simulink model "PlanarModelandControl"  (R2026a) (continued)
== system PlanarModelandControl/Planta/Dinamica do Veículo/Slip Angle == (continued)
  Dead Zone3/1 -> Sum6/1
  Gain10/1 -> Sum6/2
  Dead Zone4/1 -> Sum8/1
  Gain11/1 -> Sum8/2
  Sum5/1 -> Out Bus Element1/1  "FR"
  Atan2/1 -> Out Bus Element2/1  "RL"

== system PlanarModelandControl/Planta/Movimento do Veiculo/Movimento X ==
  note: Drag
  note: Movimento X
  Bus Element In1 : Inport  PortName=Yaw  Element=v_yaw
  BusElementIn2 : Inport  Port=2  PortName=Dinamica  Element=RollingResistances.Frx_RL
  BusElementIn3 : Inport  Port=2  PortName=Dinamica  Element=RollingResistances.Frx_RR
  BusElementIn4 : Inport  Port=2  PortName=Dinamica  Element=LatAndLongForces.Fx_RL
  BusElementIn5 : Inport  Port=2  PortName=Dinamica  Element=LatAndLongForces.Fx_RR
  BusElementIn : Inport  Port=3  PortName=ProjectedFrontForces  Element=Fxref_FL
  BusElementIn6 : Inport  Port=3  PortName=ProjectedFrontForces  Element=Fxref_FR
  Vy_cg : Inport  Port=4
  Gain1 : Gain  Gain=1/M
  Gain8 : Gain  Gain=0.5*rhoar*A*Cd
  Integrator : DiscreteIntegrator  InitialCondition=Vx_init  SampleTime=-1  ICPrevOutput=DiscIntNeverNeededParam  ICPrevScaledInput=DiscIntNeverNeededParam  mask(DiscretizedDiscreteIntegrator){}
      mask init:
      | %zoh
  Product : Product
  Square : Math  Operator=square
  Sum3 : Sum  Inputs=|++
  Sum4 : Sum  Inputs=|+--
  Sum5 : Sum  Inputs=|++
  Sum7 : Sum  Inputs=|++++
  Ax_cg : Outport
  Vx_cg : Outport  Port=2
  wires:
  Gain1/1 -> Ax_cg/1, Sum5/1  "Ax_Cg"
  Sum7/1 -> Sum4/1  "Forças de Tração"
  BusElementIn2/1 -> Sum3/1
  Sum3/1 -> Sum4/2  "resistivas"
  BusElementIn/1 -> Sum7/1
  Vy_cg/1 -> Product/1
  Product/1 -> Sum5/2  "yaw"
  BusElementIn6/1 -> Sum7/2
  Sum4/1 -> Gain1/1
  BusElementIn5/1 -> Sum7/4
  BusElementIn4/1 -> Sum7/3
  BusElementIn3/1 -> Sum3/2
  Gain8/1 -> Sum4/3
  Integrator/1 -> Square/1, Vx_cg/1  "Vx_cg"
  Bus Element In1/1 -> Product/2
  Sum5/1 -> Integrator/1  "Ax"
  Square/1 -> Gain8/1

== system PlanarModelandControl/Planta/Movimento do Veiculo/Movimento Y ==
  note: Movimento Y
  In Bus Element : Inport  PortName=ProjectedFrontForces  Element=Fyref_FL
  In Bus Element1 : Inport  PortName=ProjectedFrontForces  Element=Fyref_FR
  BusElementIn : Inport  Port=2  PortName=Dinamica  Element=LatAndLongForces.Fy_RL
  BusElementIn1 : Inport  Port=2  PortName=Dinamica  Element=LatAndLongForces.Fy_RR
  Bus Element In2 : Inport  Port=3  PortName=Yaw  Element=v_yaw
  Vx_cg : Inport  Port=4
  Dead Zone : DeadZone  LowerValue=-0.1  UpperValue=0.1
  Gain : Gain  Gain=1/M
  Integrator1 : DiscreteIntegrator  SampleTime=-1  ICPrevOutput=DiscIntNeverNeededParam  ICPrevScaledInput=DiscIntNeverNeededParam  mask(DiscretizedDiscreteIntegrator){}
      mask init:
      | %zoh
  Product1 : Product
  Sum : Sum  Inputs=|++++
  Sum6 : Sum  Inputs=|+-
  Ay_cg : Outport
  Vy_cg : Outport  Port=2
  wires:
  Sum/1 -> Gain/1
  In Bus Element1/1 -> Sum/2
  In Bus Element/1 -> Sum/1
  BusElementIn1/1 -> Sum/4
  BusElementIn/1 -> Sum/3
  Dead Zone/1 -> Product1/2
  Product1/1 -> Sum6/2  "Yaw"
  Integrator1/1 -> Vy_cg/1  "Vy_cg"
  Sum6/1 -> Integrator1/1  "Ay_cg"
  Vx_cg/1 -> Product1/1
  Gain/1 -> Sum6/1, Ay_cg/1  "Ay_cg"
  Bus Element In2/1 -> Dead Zone/1

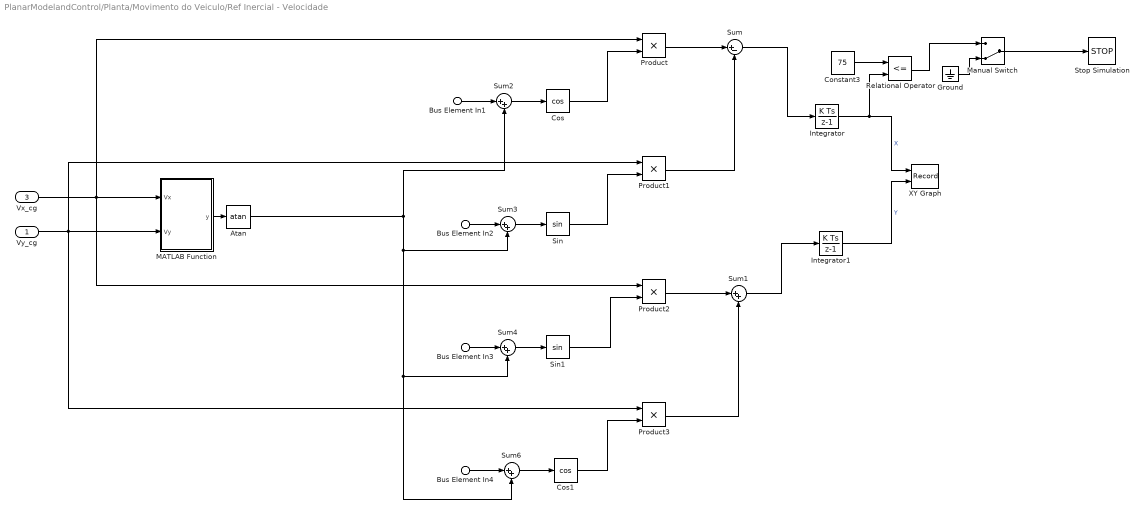
== system PlanarModelandControl/Planta/Movimento do Veiculo/Ref Inercial - Velocidade ==
  Vy_cg : Inport
  Bus Element In{1..4} (4 alike) : Inport  Port=2  PortName=Yaw  Element=s_yaw
  Vx_cg : Inport  Port=3
  Atan : Trigonometry  Operator=atan
  Constant3 : Constant  Value=75
  Cos : Trigonometry  Operator=cos
  Cos1 : Trigonometry  Operator=cos
  Ground : Ground
  Integrator : DiscreteIntegrator  SampleTime=-1  ICPrevOutput=DiscIntNeverNeededParam  ICPrevScaledInput=DiscIntNeverNeededParam  mask(DiscretizedDiscreteIntegrator){}
      mask init:
      | %zoh
  Integrator1 : DiscreteIntegrator  SampleTime=-1  ICPrevOutput=DiscIntNeverNeededParam  ICPrevScaledInput=DiscIntNeverNeededParam  mask(DiscretizedDiscreteIntegrator){}
      mask init:
      | %zoh
  MATLAB Function : MATLAB Function  TreatAsAtomicUnit=on
  Manual Switch : ManualSwitch  CurrentSetting=0
  Product{,1..3} (4 alike) : Product
  Relational Operator : RelationalOperator  Operator=<=  OutDataTypeStr=boolean  RndMeth=Simplest  InputSameDT=off
  Sin : Trigonometry
  Sin1 : Trigonometry
  Stop Simulation : Stop
  Sum : Sum  Inputs=|+-
  Sum{1..4,6} (5 alike) : Sum  Inputs=|++
  XY Graph : Record  NumPorts=2.0  FileSettingsInternal="{\"entries\":[{\"content\":{\"excelSettings\":{\"content\":{},\"type\":\"Streamout.ExcelSettings\"},\"parquetSettings\":{\"content\":{},\"type\":\"Streamout.ParquetSettings\"}},\"type\":\"Streamout.FileSettingStorage\",\"uuid\":\"\"}],\"packageUris\":[\"http:\\/\\/schema.mathworks.com\\/mf0\\/streamout_blocks\\/R2020a\"],\"version\":\"1.0\"}"  FrameSettings=[0, 0]  Layout=[1 1]  SignalsInternal="{\"entries\":[{\"content\":{\"signals\":[{\"content\":{\"blockPath\":[],\"dimensions\":[1],\"domain\":\"\",\"lineColor\":\"#7e2f8e\",\"lineWidth\":2,\"port\":1,\"sid\":[\"\"],\"signalID\":1,\"signalName\":\"X\"},\"type\":\"RecordBlkView.Signal\",\"uuid\":\"\"},{\"content\":{\"blockPath\":[],\"dimensions\":[1],\"domain\":\"\",\"lineColor\":\"#77ac30\",\"lineWidth\":2,\"port\":2,\"sid\":[\"\"],\"signalID\":2,\"signalName\":\"Y\"},\"type\":\"RecordBlkView.Signal\",\"uuid\":\"\"}]},\"type\":\"RecordBlkView.InputSignals\",\"uuid\":\"\"}],\"packageUris\":[\"http:\\/\\/schema.mathworks.com\\/mf0\\/streamout_blocks\\/R2020a\"],\"version\":\"1.0\"}"  VisualizationBindings="{\"xyplotplugin\":{\"info\":[{\"series\":[],\"subplotID\":1}]}}"  CompatibilityTag=XY  st=-1
  wires:
  Cos/1 -> Product/2
  Product/1 -> Sum/1
  Sin/1 -> Product1/2
  Product1/1 -> Sum/2
  Sum/1 -> Integrator/1
  Sin1/1 -> Product2/2
  Product2/1 -> Sum1/1
  Product3/1 -> Sum1/2
  Cos1/1 -> Product3/2
  Sum1/1 -> Integrator1/1
  Integrator1/1 -> XY Graph/2  "Y"
  Integrator/1 -> Relational Operator/2, XY Graph/1  "X"
  Bus Element In1/1 -> Sum2/1
  Bus Element In2/1 -> Sum3/1
  Bus Element In3/1 -> Sum4/1
  Bus Element In4/1 -> Sum6/1
  Vx_cg/1 -> Product/1, Product2/1, MATLAB Function/1(Vx)
  Vy_cg/1 -> Product3/1, Product1/1, MATLAB Function/2(Vy)
  MATLAB Function/1(y) -> Atan/1
  Sum2/1 -> Cos/1
  Sum3/1 -> Sin/1
  Atan/1 -> Sum2/2, Sum6/2, Sum4/2, Sum3/2
  Sum6/1 -> Cos1/1
  Sum4/1 -> Sin1/1
  Constant3/1 -> Relational Operator/1
  Relational Operator/1 -> Manual Switch/1
  Ground/1 -> Manual Switch/2
  Manual Switch/1 -> Stop Simulation/1

== system PlanarModelandControl/Planta/Movimento do Veiculo/Xres ==
  note: FR
  note: FL
  BusElementIn2 : Inport  PortName=Dinamica  Element=RollingResistances.Frx_FL
  BusElementIn3 : Inport  PortName=Dinamica  Element=RollingResistances.Frx_FR
  BusElementIn : Inport  PortName=Dinamica  Element=LatAndLongForces.Fx_FL
  BusElementIn4 : Inport  PortName=Dinamica  Element=LatAndLongForces.Fy_FL
  BusElementIn5 : Inport  PortName=Dinamica  Element=LatAndLongForces.Fy_FR
  BusElementIn1 : Inport  PortName=Dinamica  Element=LatAndLongForces.Fx_FR
  Bus Element In3 : Inport  Port=2  PortName=Driver  Element=steering_outer
  Bus Element In1 : Inport  Port=2  PortName=Driver  Element=steering_inner
  Bus Element In2 : Inport  Port=2  PortName=Driver  Element=steering_inner
  Cos : Trigonometry  Operator=cos
  Cos1 : Trigonometry
  Cos2 : Trigonometry  Operator=cos
  Cos3 : Trigonometry
  Product{,1..3} (4 alike) : Product
  Sum{,1..3} (4 alike) : Sum  Inputs=|+-
  Fxref_FL : Outport
  Fxref_FR : Outport  Port=2
  wires:
  BusElementIn2/1 -> Sum/2
  Sum/1 -> Product/1
  Cos/1 -> Product/2
  Bus Element In1/1 -> Cos/1
  Product/1 -> Sum1/1
  Product1/1 -> Sum1/2
  Bus Element In2/1 -> Cos1/1
  BusElementIn4/1 -> Product1/1
  BusElementIn5/1 -> Product3/1
  Cos3/1 -> Product3/2
  Bus Element In3/1 -> Cos3/1, Cos2/1
  Product3/1 -> Sum3/2
  Product2/1 -> Sum3/1
  Cos2/1 -> Product2/2
  Sum2/1 -> Product2/1
  BusElementIn3/1 -> Sum2/2
  Cos1/1 -> Product1/2
  BusElementIn/1 -> Sum/1
  BusElementIn1/1 -> Sum2/1
  Sum1/1 -> Fxref_FL/1
  Sum3/1 -> Fxref_FR/1

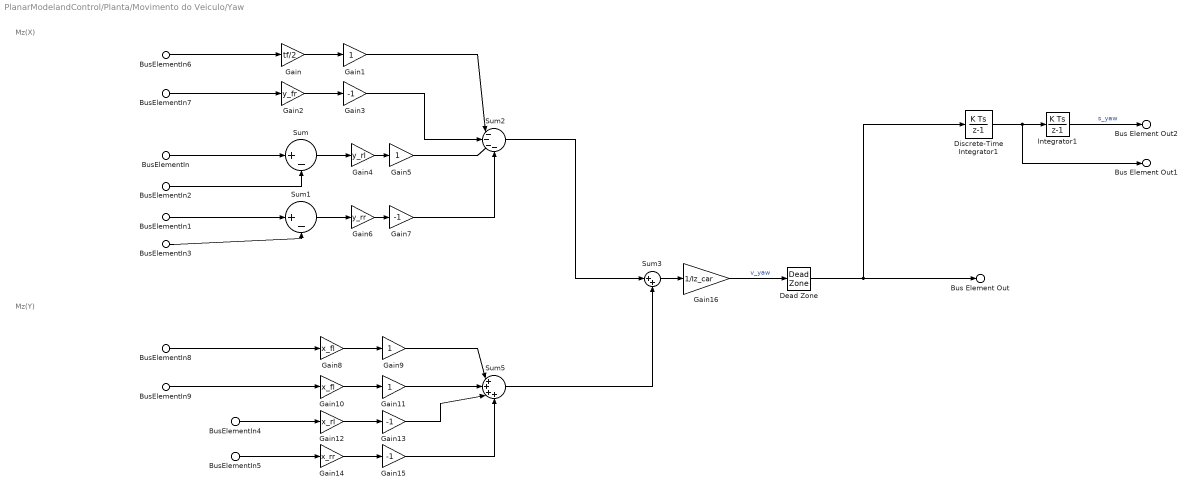
== system PlanarModelandControl/Planta/Movimento do Veiculo/Yaw ==
  note: Mz(X)
  note: Mz(Y)
  BusElementIn2 : Inport  PortName=Dinamica  Element=RollingResistances.Frx_RL
  BusElementIn3 : Inport  PortName=Dinamica  Element=RollingResistances.Frx_RR
  BusElementIn : Inport  PortName=Dinamica  Element=LatAndLongForces.Fx_RL
  BusElementIn1 : Inport  PortName=Dinamica  Element=LatAndLongForces.Fx_RR
  BusElementIn4 : Inport  PortName=Dinamica  Element=LatAndLongForces.Fy_RL
  BusElementIn5 : Inport  PortName=Dinamica  Element=LatAndLongForces.Fy_RR
  BusElementIn6 : Inport  Port=2  PortName=ProjectedFrontForces  Element=Fxref_FL
  BusElementIn7 : Inport  Port=2  PortName=ProjectedFrontForces  Element=Fxref_FR
  BusElementIn8 : Inport  Port=2  PortName=ProjectedFrontForces  Element=Fyref_FL
  BusElementIn9 : Inport  Port=2  PortName=ProjectedFrontForces  Element=Fyref_FR
  Dead Zone : DeadZone  LowerValue=-0.1  UpperValue=0.1
  Discrete-Time Integrator1 : DiscreteIntegrator  SampleTime=-1  ICPrevOutput=DiscIntNeverNeededParam  ICPrevScaledInput=DiscIntNeverNeededParam
  Gain : Gain  Gain=tf/2
  Gain{1,5,9,11} (4 alike) : Gain
  Gain10 : Gain  Gain=x_fl
  Gain12 : Gain  Gain=x_rl
  Gain{3,7,13,15} (4 alike) : Gain  Gain=-1
  Gain14 : Gain  Gain=x_rr
  Gain16 : Gain  Gain=1/Iz_car
  Gain2 : Gain  Gain=y_fr
  Gain4 : Gain  Gain=y_rl
  Gain6 : Gain  Gain=y_rr
  Gain8 : Gain  Gain=x_fl
  Integrator1 : DiscreteIntegrator  SampleTime=-1  ICPrevOutput=DiscIntNeverNeededParam  ICPrevScaledInput=DiscIntNeverNeededParam  mask(DiscretizedDiscreteIntegrator){}
      mask init:
      | %zoh
  Sum : Sum  Inputs=|+-
  Sum1 : Sum  Inputs=|+-
  Sum2 : Sum  Inputs=|----
  Sum3 : Sum  Inputs=|++
  Sum5 : Sum  Inputs=|++++
  Bus Element Out : Outport  VectorParamsAs1DForOutWhenUnconnected=off  PortName=Yaw  Element=a_yaw
  Bus Element Out1 : Outport  VectorParamsAs1DForOutWhenUnconnected=off  PortName=Yaw  Element=v_yaw
  Bus Element Out2 : Outport  VectorParamsAs1DForOutWhenUnconnected=off  PortName=Yaw  Element=s_yaw
  wires:
  BusElementIn3/1 -> Sum1/2
  BusElementIn2/1 -> Sum/2
  Gain/1 -> Gain1/1
  Gain2/1 -> Gain3/1
  Sum/1 -> Gain4/1
  Gain4/1 -> Gain5/1
  Sum1/1 -> Gain6/1
  Gain6/1 -> Gain7/1
  Gain1/1 -> Sum2/1
  Gain3/1 -> Sum2/2
  Gain5/1 -> Sum2/3
  Gain15/1 -> Sum5/4
  Gain13/1 -> Sum5/3
  Gain11/1 -> Sum5/2
  Gain9/1 -> Sum5/1
  Gain14/1 -> Gain15/1
  Gain12/1 -> Gain13/1
  Gain10/1 -> Gain11/1
  Gain8/1 -> Gain9/1
  Sum2/1 -> Sum3/1
  Sum5/1 -> Sum3/2
  Sum3/1 -> Gain16/1
  Gain16/1 -> Dead Zone/1  "v_yaw"
  Integrator1/1 -> Bus Element Out2/1  "s_yaw"
  BusElementIn/1 -> Sum/1
  BusElementIn1/1 -> Sum1/1
  BusElementIn5/1 -> Gain14/1
  BusElementIn4/1 -> Gain12/1
  Gain7/1 -> Sum2/4
  Discrete-Time Integrator1/1 -> Integrator1/1, Bus Element Out1/1
  Dead Zone/1 -> Bus Element Out/1, Discrete-Time Integrator1/1
  BusElementIn6/1 -> Gain/1
  BusElementIn7/1 -> Gain2/1
  BusElementIn8/1 -> Gain8/1
  BusElementIn9/1 -> Gain10/1

== system PlanarModelandControl/Planta/Movimento do Veiculo/Yres ==
  note: FL
  note: FL
  Bus Element In1 : Inport  PortName=Driver  Element=steering_inner
  Bus Element In2 : Inport  PortName=Driver  Element=steering_outer
  BusElementIn : Inport  Port=2  PortName=Dinamica  Element=RollingResistances.Frx_FL
  BusElementIn1 : Inport  Port=2  PortName=Dinamica  Element=RollingResistances.Frx_FR
  BusElementIn2 : Inport  Port=2  PortName=Dinamica  Element=LatAndLongForces.Fx_FL
  BusElementIn3 : Inport  Port=2  PortName=Dinamica  Element=LatAndLongForces.Fx_FR
  BusElementIn4 : Inport  Port=2  PortName=Dinamica  Element=LatAndLongForces.Fy_FL
  BusElementIn5 : Inport  Port=2  PortName=Dinamica  Element=LatAndLongForces.Fy_FR
  Cos : Trigonometry
  Cos1 : Trigonometry  Operator=cos
  Cos2 : Trigonometry
  Cos3 : Trigonometry  Operator=cos
  Product{,1..3} (4 alike) : Product
  Sum : Sum  Inputs=|+-
  Sum1 : Sum  Inputs=|++
  Sum2 : Sum  Inputs=|+-
  Sum3 : Sum  Inputs=|++
  Fyref_FL : Outport
  Fyref_FR : Outport  Port=2
  wires:
  Product1/1 -> Sum1/2
  Cos1/1 -> Product1/2
  Bus Element In1/1 -> Cos1/1, Cos/1
  Sum/1 -> Product/1
  Cos/1 -> Product/2
  Product/1 -> Sum1/1
  Product2/1 -> Sum3/1
  Cos2/1 -> Product2/2
  Sum2/1 -> Product2/1
  BusElementIn1/1 -> Sum2/2
  Bus Element In2/1 -> Cos3/1, Cos2/1
  Cos3/1 -> Product3/2
  Product3/1 -> Sum3/2
  BusElementIn4/1 -> Product1/1
  BusElementIn5/1 -> Product3/1
  BusElementIn3/1 -> Sum2/1
  Sum3/1 -> Fyref_FR/1
  Sum1/1 -> Fyref_FL/1
  BusElementIn/1 -> Sum/2
  BusElementIn2/1 -> Sum/1

== system PlanarModelandControl/Controle/Direct Yaw Control/MDYC/Feedback ==
  note: Limite inf e sup do motor
  Yaw rate : Inport
  Vx : Inport  Port=2
  Yaw_des : Inport  Port=3
  Pedal map : Inport  Port=4
  Gain : Gain  Gain=Ts
  KI : Product
  KP : Product
  Limite min : Lookup_n-D  NumberOfTableDimensions=1  BreakpointsForDimension1=pedal  BreakpointsForDimension3=[5, 7]  Table=Mz_minF  InternalRulePriority=Speed  RndMeth=Simplest
  Limite máx : Lookup_n-D  NumberOfTableDimensions=1  BreakpointsForDimension1=pedal  BreakpointsForDimension3=[5, 7]  Table=Mz_maxF  InternalRulePriority=Speed  RndMeth=Simplest
  Saturation Dynamic : sl_discontinuities/Saturation Dynamic  OutMin=[]  OutMax=[]  OutDataTypeStr=Inherit: Same as second input  OutDataType=fixdt(1, 16)  OutScaling=2^-10  DoSatur=off
  Sum : Sum  Inputs=|+-
  Sum1 : Sum  Inputs=|++
  Sum2 : Sum  Inputs=|++
  Unit Delay : UnitDelay  SampleTime=-1
  n-D Lookup Table : Lookup_n-D  NumberOfTableDimensions=1  BreakpointsForDimension1=Vx_axis  BreakpointsForDimension3=[5, 7]  Table=Kp_data  InternalRulePriority=Speed  RndMeth=Simplest
  n-D Lookup Table1 : Lookup_n-D  NumberOfTableDimensions=1  BreakpointsForDimension1=Vx_axis  BreakpointsForDimension3=[5, 7]  Table=Ki_data  InternalRulePriority=Speed  RndMeth=Simplest
  Mz_FB : Outport
  wires:
  Pedal map/1 -> Limite min/1, Limite máx/1
  Limite máx/1 -> Saturation Dynamic/1
  Limite min/1 -> Saturation Dynamic/3
  Yaw_des/1 -> Sum/1  "Yaw_Des"
  Yaw rate/1 -> Sum/2  "Yaw_rate"
  n-D Lookup Table/1 -> KP/2
  n-D Lookup Table1/1 -> KI/2
  Sum/1 -> KI/1, KP/1
  KI/1 -> Gain/1
  Gain/1 -> Sum1/2  "Ki"
  Unit Delay/1 -> Sum1/1
  Sum1/1 -> Sum2/2, Unit Delay/1
  KP/1 -> Sum2/1
  Vx/1 -> n-D Lookup Table1/1, n-D Lookup Table/1
  Sum2/1 -> Saturation Dynamic/2
  Saturation Dynamic/1 -> Mz_FB/1

== system PlanarModelandControl/Controle/Direct Yaw Control/MDYC/Feedfoward ==
  note: Limite inf e sup do motor
  CornR : Inport
  CornF : Inport  Port=2
  K delta : Inport  Port=3
  Ay : Inport  Port=4
  Pedal map : Inport  Port=5
  Add : Sum
  Divide : Product  Inputs=*/
  Gain : Gain  Gain=2*L
  Limite min : Lookup_n-D  NumberOfTableDimensions=1  BreakpointsForDimension1=pedal  BreakpointsForDimension3=[5, 7]  Table=Mz_minF  InternalRulePriority=Speed  RndMeth=Simplest
  Limite máx : Lookup_n-D  NumberOfTableDimensions=1  BreakpointsForDimension1=pedal  BreakpointsForDimension3=[5, 7]  Table=Mz_maxF  InternalRulePriority=Speed  RndMeth=Simplest
  Product : Product
  Product1 : Product
  Product2 : Product
  Saturation Dynamic : sl_discontinuities/Saturation Dynamic  OutMin=[]  OutMax=[]  OutDataTypeStr=Inherit: Same as second input  OutDataType=fixdt(1, 16)  OutScaling=2^-10  DoSatur=off
  Mz_FF : Outport
  wires:
  Limite min/1 -> Saturation Dynamic/3
  Pedal map/1 -> Limite min/1, Limite máx/1
  Limite máx/1 -> Saturation Dynamic/1
  CornR/1 -> Add/1, Product/1
  CornF/1 -> Product/2, Add/2
  Product/1 -> Gain/1
  Gain/1 -> Divide/1
  Add/1 -> Divide/2
  Divide/1 -> Product1/1
  K delta/1 -> Product1/2
  Product1/1 -> Product2/1
  Product2/1 -> Saturation Dynamic/2
  Saturation Dynamic/1 -> Mz_FF/1
  Ay/1 -> Product2/2

== system PlanarModelandControl/Controle/Direct Yaw Control/ON//OFF/If Action Subsystem ==
  Mz_ajustado : Inport
  Action Port : ActionPort  ActionPortLabel=if(u1<0.05 | u2<1 | u3<2 | u4>0)
  Gain : Gain  Gain=0
  Mz_OFF : Outport
  wires:
  Mz_ajustado/1 -> Gain/1
  Gain/1 -> Mz_OFF/1

== system PlanarModelandControl/Controle/Direct Yaw Control/ON//OFF/If Action Subsystem1 ==
  Mz_ajustado : Inport
  Action Port : ActionPort  ActionPortLabel=else
  Mz_ON : Outport
  wires:
  Mz_ajustado/1 -> Mz_ON/1

== system PlanarModelandControl/Controle/Direct Yaw Control/Yaw desejado/ref < limit ==
  Yaw_ref : Inport
  Action Port : ActionPort  ActionPortLabel=else
  Yaw_des : Outport
  wires:
  Yaw_ref/1 -> Yaw_des/1

== system PlanarModelandControl/Controle/Direct Yaw Control/Yaw desejado/ref > limit ==
  Yaw_limit : Inport
  Yaw_ref : Inport  Port=2
  Action Port : ActionPort  ActionPortLabel=if(u2 > u1)
  Product : Product
  Sign : Signum
  Yaw_des : Outport
  wires:
  Yaw_ref/1 -> Sign/1
  Yaw_limit/1 -> Product/1
  Sign/1 -> Product/2
  Product/1 -> Yaw_des/1

== system PlanarModelandControl/Controle/Traction Control/TC_FL/Fuzzy Controller ==
  wheel slip : Inport
  Discrete Derivative1 : simulink/Discrete/Discrete Derivative  gainval=1.0  ICPrevScaledInput=0.0  OutMin=[]  OutMax=[]  OutDataType=fixdt(1, 16)  OutScaling=2^-10  DoSatur=off
  Fuzzy Logic Controller : fuzblock/Fuzzy Logic  Controller  FIS='tc6.fis'  OutputSampleNumber=101  DataType=double  UseLookupTable=off  NumBreakpoints=10  FuzzifiedInputs=off  RuleFiringStrengths=off  RuleOutputs=off  AggregatedOutputs=off  SimulateUsing=Code generation  OutOfRangeInputValueMessage=warning  NoRuleFiredMessage=warning  EmptyOutputFuzzySetMessage=warning
  Mux : Mux  Inputs=2
  Saturation : Saturate  UpperLimit=1  LowerLimit=0
  Torque reducing coefficient : Outport
  wires:
  Mux/1 -> Fuzzy Logic Controller/1
  Fuzzy Logic Controller/1 -> Saturation/1
  Saturation/1 -> Torque reducing coefficient/1
  Discrete Derivative1/1 -> Mux/2
  wheel slip/1 -> Mux/1, Discrete Derivative1/1

== system PlanarModelandControl/Controle/Traction Control/TC_FR/Fuzzy Controller ==
  wheel slip : Inport
  Discrete Derivative1 : simulink/Discrete/Discrete Derivative  gainval=1.0  ICPrevScaledInput=0.0  OutMin=[]  OutMax=[]  OutDataType=fixdt(1, 16)  OutScaling=2^-10  DoSatur=off
  Fuzzy Logic Controller : fuzblock/Fuzzy Logic  Controller  FIS='tc6.fis'  OutputSampleNumber=101  DataType=double  UseLookupTable=off  NumBreakpoints=10  FuzzifiedInputs=off  RuleFiringStrengths=off  RuleOutputs=off  AggregatedOutputs=off  SimulateUsing=Code generation  OutOfRangeInputValueMessage=warning  NoRuleFiredMessage=warning  EmptyOutputFuzzySetMessage=warning
  Mux : Mux  Inputs=2
  Saturation : Saturate  UpperLimit=1  LowerLimit=0
  Torque reducing coefficient : Outport
  wires:
  Mux/1 -> Fuzzy Logic Controller/1
  Fuzzy Logic Controller/1 -> Saturation/1
  Saturation/1 -> Torque reducing coefficient/1
  Discrete Derivative1/1 -> Mux/2
  wheel slip/1 -> Mux/1, Discrete Derivative1/1

== system PlanarModelandControl/Controle/Traction Control/TC_RL/Fuzzy Controller ==
  wheel slip : Inport
  Discrete Derivative1 : simulink/Discrete/Discrete Derivative  gainval=1.0  ICPrevScaledInput=0.0  OutMin=[]  OutMax=[]  OutDataType=fixdt(1, 16)  OutScaling=2^-10  DoSatur=off
  Fuzzy Logic Controller : fuzblock/Fuzzy Logic  Controller  FIS='tc6.fis'  OutputSampleNumber=101  DataType=double  UseLookupTable=off  NumBreakpoints=10  FuzzifiedInputs=off  RuleFiringStrengths=off  RuleOutputs=off  AggregatedOutputs=off  SimulateUsing=Code generation  OutOfRangeInputValueMessage=warning  NoRuleFiredMessage=warning  EmptyOutputFuzzySetMessage=warning
  Mux : Mux  Inputs=2
  Saturation : Saturate  UpperLimit=1  LowerLimit=0
  Torque reducing coefficient : Outport
  wires:
  Mux/1 -> Fuzzy Logic Controller/1
  Fuzzy Logic Controller/1 -> Saturation/1
  Saturation/1 -> Torque reducing coefficient/1
  Discrete Derivative1/1 -> Mux/2
  wheel slip/1 -> Mux/1, Discrete Derivative1/1

== system PlanarModelandControl/Controle/Traction Control/TC_RR/Fuzzy Controller ==
  wheel slip : Inport
  Discrete Derivative1 : simulink/Discrete/Discrete Derivative  gainval=1.0  ICPrevScaledInput=0.0  OutMin=[]  OutMax=[]  OutDataType=fixdt(1, 16)  OutScaling=2^-10  DoSatur=off
  Fuzzy Logic Controller : fuzblock/Fuzzy Logic  Controller  FIS='tc6.fis'  OutputSampleNumber=101  DataType=double  UseLookupTable=off  NumBreakpoints=10  FuzzifiedInputs=off  RuleFiringStrengths=off  RuleOutputs=off  AggregatedOutputs=off  SimulateUsing=Code generation  OutOfRangeInputValueMessage=warning  NoRuleFiredMessage=warning  EmptyOutputFuzzySetMessage=warning
  Mux : Mux  Inputs=2
  Saturation : Saturate  UpperLimit=1  LowerLimit=0
  Torque reducing coefficient : Outport
  wires:
  Mux/1 -> Fuzzy Logic Controller/1
  Fuzzy Logic Controller/1 -> Saturation/1
  Saturation/1 -> Torque reducing coefficient/1
  Discrete Derivative1/1 -> Mux/2
  wheel slip/1 -> Mux/1, Discrete Derivative1/1

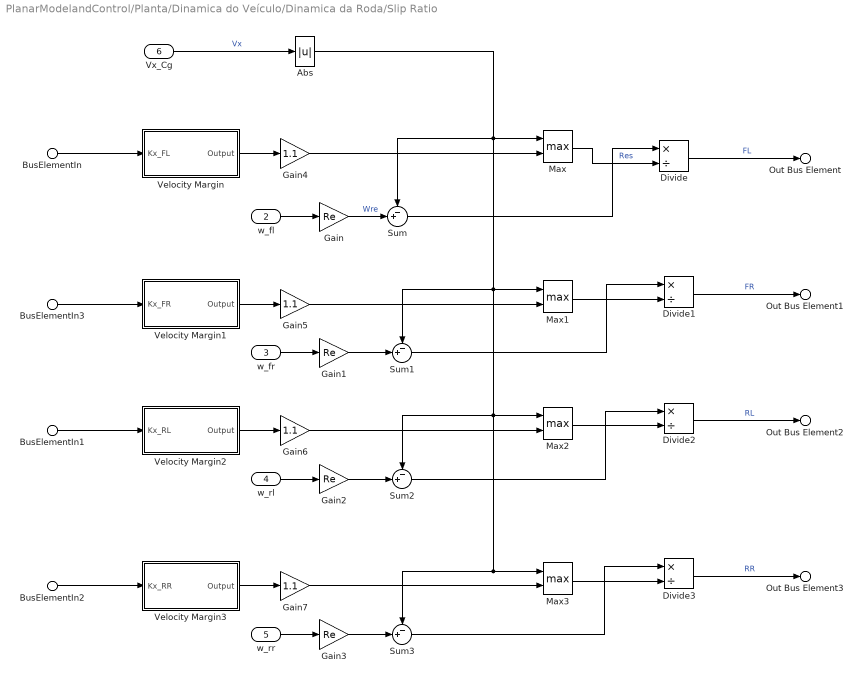
== system PlanarModelandControl/Planta/Dinamica do Veículo/Dinamica da Roda/Slip Ratio ==
  BusElementIn : Inport  PortName=Pacejka  Element=Kx_FL
  BusElementIn1 : Inport  PortName=Pacejka  Element=Kx_RL
  BusElementIn2 : Inport  PortName=Pacejka  Element=Kx_RR
  BusElementIn3 : Inport  PortName=Pacejka  Element=Kx_FR
  w_fl : Inport  Port=2
  w_fr : Inport  Port=3
  w_rl : Inport  Port=4
  w_rr : Inport  Port=5
  Vx_Cg : Inport  Port=6
  Abs : Abs  SaturateOnIntegerOverflow=off
  Divide{,1..3} (4 alike) : Product  Inputs=*/
  Gain{,1..3} (4 alike) : Gain  Gain=Re
  Gain{4..7} (4 alike) : Gain  Gain=1.1
  Max{,1..3} (4 alike) : MinMax  Function=max  Inputs=2
  Sum{,1..3} (4 alike) : Sum  Inputs=-+|
  Velocity Margin : Subsystem
  Velocity Margin1 : Subsystem
  Velocity Margin2 : Subsystem
  Velocity Margin3 : Subsystem
  Out Bus Element : Outport  PortName=WheelSlips  Element=slip_long_FL
  Out Bus Element3 : Outport  PortName=WheelSlips  Element=slip_long_RR
  Out Bus Element2 : Outport  PortName=WheelSlips  Element=slip_long_RL
  Out Bus Element1 : Outport  PortName=WheelSlips  Element=slip_long_FR
  wires:
  w_fl/1 -> Gain/1
  w_fr/1 -> Gain1/1
  w_rl/1 -> Gain2/1
  w_rr/1 -> Gain3/1
  Gain2/1 -> Sum2/2
  Vx_Cg/1 -> Abs/1  "Vx"
  Sum2/1 -> Divide2/1
  Max1/1 -> Divide1/2
  Max2/1 -> Divide2/2
  Max3/1 -> Divide3/2
  Velocity Margin/1(Output) -> Gain4/1
  Velocity Margin1/1(Output) -> Gain5/1
  Velocity Margin2/1(Output) -> Gain6/1
  Velocity Margin3/1(Output) -> Gain7/1
  Max/1 -> Divide/2  "Res"
  Sum3/1 -> Divide3/1
  Gain3/1 -> Sum3/2
  Sum/1 -> Divide/1
  Sum1/1 -> Divide1/1
  Gain5/1 -> Max1/2
  Gain4/1 -> Max/2
  Abs/1 -> Sum3/1, Max3/1, Sum2/1, Max2/1, Sum1/1, Max1/1, Sum/1, Max/1
  Gain7/1 -> Max3/2
  Gain6/1 -> Max2/2
  Gain1/1 -> Sum1/2
  Gain/1 -> Sum/2  "Wre"
  Divide/1 -> Out Bus Element/1  "FL"
  Divide1/1 -> Out Bus Element1/1  "FR"
  Divide2/1 -> Out Bus Element2/1  "RL"
  Divide3/1 -> Out Bus Element3/1  "RR"
  BusElementIn/1 -> Velocity Margin/1(Kx_FL)
  BusElementIn3/1 -> Velocity Margin1/1(Kx_FR)
  BusElementIn1/1 -> Velocity Margin2/1(Kx_RL)
  BusElementIn2/1 -> Velocity Margin3/1(Kx_RR)

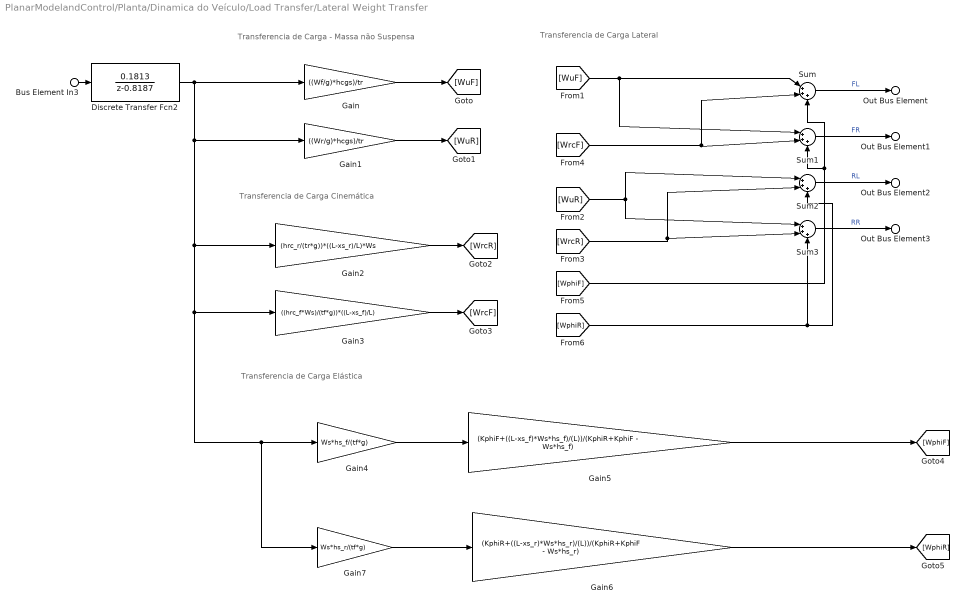
== system PlanarModelandControl/Planta/Dinamica do Veículo/Load Transfer/Lateral Weight Transfer ==
  note: Transferencia de Carga Elástica
  note: Transferencia de Carga - Massa não Suspensa
  note: Transferencia de Carga Cinemática
  note: Transferencia de Carga Lateral
  Bus Element In3 : Inport  PortName=Movimento  Element=Ay_cg
  Discrete Transfer Fcn2 : DiscreteTransferFcn  Numerator=[0.1813]  Denominator="[1  -0.8187]"
  Gain : Gain  Gain=((Wf/g)*hcgs)/tr
  Gain1 : Gain  Gain=((Wr/g)*hcgs)/tr
  Gain2 : Gain  Gain=(hrc_r/(tr*g))*((L-xs_r)/L)*Ws
  Gain3 : Gain  Gain=((hrc_f*Ws)/(tf*g))*((L-xs_f)/L)
  Gain4 : Gain  Gain=Ws*hs_f/(tf*g)
  Gain5 : Gain  Gain=(KphiF+((L-xs_f)*Ws*hs_f)/(L))/(KphiR+KphiF - Ws*hs_f)
  Gain6 : Gain  Gain=(KphiR+((L-xs_r)*Ws*hs_r)/(L))/(KphiR+KphiF - Ws*hs_r)
  Gain7 : Gain  Gain=Ws*hs_r/(tf*g)
  Sum{,1..3} (4 alike) : Sum  Inputs=|+++
  Out Bus Element : Outport  PortName=DeltaW_lat  Element=WLR_FL
  Out Bus Element1 : Outport  PortName=DeltaW_lat  Element=WLR_FR
  Out Bus Element2 : Outport  PortName=DeltaW_lat  Element=WLR_RL
  Out Bus Element3 : Outport  PortName=DeltaW_lat  Element=WLR_RR
  wires:
  Sum/1 -> Out Bus Element/1  "FL"
  Gain1/1 -> [WuR]
  Gain6/1 -> [WphiR]
  [WphiR] -> Sum3/3, Sum2/3
  Gain3/1 -> [WrcF]
  [WrcF] -> Sum1/2, Sum/2
  Gain7/1 -> Gain6/1
  Sum3/1 -> Out Bus Element3/1  "RR"
  [WuF] -> Sum1/1, Sum/1
  Gain5/1 -> [WphiF]
  [WrcR] -> Sum2/2, Sum3/2
  [WuR] -> Sum2/1, Sum3/1
  Gain2/1 -> [WrcR]
  Bus Element In3/1 -> Discrete Transfer Fcn2/1
  Gain4/1 -> Gain5/1
  [WphiF] -> Sum/3, Sum1/3
  Discrete Transfer Fcn2/1 -> Gain/1, Gain7/1, Gain4/1, Gain3/1, Gain2/1, Gain1/1
  Gain/1 -> [WuF]
  Sum1/1 -> Out Bus Element1/1  "FR"
  Sum2/1 -> Out Bus Element2/1  "RL"
